simulink model "vit_tiny_simulink_v2"  (R2026a)
settings: Solver=FixedStepAuto
model workspace (MAT data in the model file):
  b0_bout = [0.778968453407 -0.0598331429064 0.518007159233 -0.0489355251193 -0.120259530842 -0.233932569623 0.145202979445 -0.609933674335 0.0480090156198 0.108426816761 -0.264556109905 0.0985349565744 ... (192 elements, 192x1)]
  b0_bqkv = [0.646790981293 1.11617136002 0.681947648525 0.380520701408 -0.228939250112 -0.210620775819 0.443939357996 0.168313741684 -0.0972141698003 0.138269111514 0.0691619515419 -0.232958853245 ... (576 elements, 576x1)]
  b0_wout = [-0.00644482811913 -0.0429702401161 0.0362374633551 -0.0623319223523 0.0345713756979 0.0856178253889 -0.087087020278 -0.0191561747342 -0.00712048402056 -0.00242649461143 -0.0159777421504 0.0877591148019 ... (36864 elements, 192x192)]
  b0_wqkv = [-0.111820198596 -0.061643447727 0.054388653487 -0.00236934260465 -0.124385409057 -0.0232415217906 -0.144188866019 0.097465723753 0.105003915727 -0.00340389972553 -0.123615674675 0.0953903868794 ... (110592 elements, 576x192)]
  b10_bout = [0.0401422604918 -0.173094883561 0.0153807215393 0.0737516358495 0.101318269968 -0.102552995086 -0.291259735823 -0.224377304316 0.0514300614595 -0.027960466221 -0.0377635993063 0.0201884657145 ... (192 elements, 192x1)]
  b10_bqkv = [-0.0802283063531 -0.193966910243 0.00535996071994 -0.170352563262 0.0527183525264 0.528374612331 0.381562888622 -0.241518482566 0.914053916931 -0.215931251645 0.12868578732 0.273458838463 ... (576 elements, 576x1)]
  b10_wout = [0.0782992020249 -0.0179750658572 0.129645258188 -0.0809217840433 0.0186945144087 0.0538404919207 -0.157687038183 -0.0309003889561 -0.0224377308041 -0.0750434324145 -0.134708985686 0.0406404659152 ... (36864 elements, 192x192)]
  b10_wqkv = [-0.0681082233787 0.0488544106483 -0.0731555297971 -0.0011171658989 -0.0821463242173 0.0175136197358 -0.0203201379627 0.0517607964575 -0.0496901981533 0.0509784445167 0.0563900731504 0.0606791935861 ... (110592 elements, 576x192)]
  b11_bout = [0.731424629688 -0.672566652298 0.280835986137 0.0499101057649 0.123574219644 0.0940038487315 -0.220306441188 -0.0221516657621 -0.404082298279 0.441653996706 0.00815785769373 0.0947464555502 ... (192 elements, 192x1)]
  b11_bqkv = [-0.18868714571 0.534532427788 -0.25027307868 -0.296293616295 -0.512328565121 -0.460017681122 -4.11578655243 0.164140149951 -1.2329877615 -0.281609892845 0.52692168951 0.468302696943 ... (576 elements, 576x1)]
  b11_wout = [0.047587364912 0.000814941420685 0.0597563162446 -0.0518249236047 -0.0508356280625 -0.0792408362031 -0.074274905026 -0.165682837367 -0.121390283108 0.0330879129469 -0.0537072867155 0.0743666738272 ... (36864 elements, 192x192)]
  b11_wqkv = [-0.0458272658288 0.0325416885316 0.0669981464744 0.0917598679662 0.0799371525645 -0.0330501869321 -0.000155119312694 0.0641588270664 0.144193008542 0.102804735303 0.00301717664115 0.0656418129802 ... (110592 elements, 576x192)]
  b1_bout = [-0.00971997715533 -0.00772349210456 -0.31704044342 0.238263577223 0.409832179546 0.283682137728 -0.0330189839005 -0.768799006939 -0.110244117677 -0.188653618097 -0.177126094699 0.0168285723776 ... (192 elements, 192x1)]
  b1_bqkv = [-0.44452881813 -0.77194994688 -0.318848788738 0.471629351377 -0.239391535521 -0.409112393856 -0.207066968083 0.650474190712 -0.0192827414721 0.194389820099 0.763711333275 -0.494900196791 ... (576 elements, 576x1)]
  b1_wout = [0.0807484909892 -0.00597395701334 -0.0312148649246 0.0136822443455 -0.03304643929 0.0206444934011 0.0792439058423 0.00468857167289 -0.025502268225 0.0522494241595 -0.0267972294241 -0.0434053950012 ... (36864 elements, 192x192)]
  b1_wqkv = [0.0512335635722 0.0885934457183 -0.00560034625232 0.00362458731979 -0.00930483732373 -0.0819085016847 0.0552942492068 -0.0290139894933 -0.0371151119471 -0.0522247776389 -0.0766076669097 -0.0357538573444 ... (110592 elements, 576x192)]
  b2_bout = [0.186909124255 -0.0196638796479 -0.0815877616405 -0.0520006269217 -0.0499247051775 -0.000725046906155 0.140784710646 -0.149694025517 0.0473203398287 0.0851504653692 -0.0695137456059 0.124424636364 ... (192 elements, 192x1)]
  b2_bqkv = [-0.0184468366206 -0.33409678936 0.0544146075845 -0.539437711239 0.0207475852221 -0.569913327694 0.675430297852 -0.0306138265878 0.137923911214 0.132708072662 -0.693137049675 -0.898855745792 ... (576 elements, 576x1)]
  b2_wout = [-0.0786214545369 -0.0264880564064 0.021444587037 -0.0203547999263 0.0681303590536 -0.0475320294499 -0.0878006964922 -0.117818422616 -0.0165466293693 -0.0438001379371 0.0672394111753 0.00145982671529 ... (36864 elements, 192x192)]
  b2_wqkv = [-0.138439133763 -0.164019718766 0.0208436828107 -0.0225581023842 0.0665642395616 0.0249229986221 -0.0014197943965 0.0313837975264 -0.118816934526 0.0859390795231 -0.0156196895987 0.0889494940639 ... (110592 elements, 576x192)]
  b3_bout = [0.0302332583815 -0.078589104116 0.0591629892588 0.122181482613 0.125220179558 0.174787655473 0.0698654949665 -0.119789279997 -0.0854825153947 0.0111166592687 -0.0519489310682 -0.0854979902506 ... (192 elements, 192x1)]
  b3_bqkv = [0.00970917753875 -0.137848749757 0.247470095754 0.00317194242962 -0.396429866552 -0.026160268113 -0.0386148132384 0.0218217838556 0.0342304669321 -0.283184826374 -0.23029743135 0.0519815944135 ... (576 elements, 576x1)]
  b3_wout = [-0.0932515189052 -0.0723011493683 -0.00572068244219 -0.0543470866978 -0.104117572308 -0.0373927094042 -0.0112823974341 0.0223814100027 0.0500929839909 -0.0560720935464 0.0953728854656 0.0339839868248 ... (36864 elements, 192x192)]
  b3_wqkv = [0.00368765974417 0.00305238785222 -0.026172157377 -0.0679289996624 -0.106250740588 -0.0884887948632 0.0913795605302 -0.0699781402946 -0.0334121137857 0.042931098491 0.0546709150076 -0.0805031657219 ... (110592 elements, 576x192)]
  b4_bout = [0.23899422586 -0.000535868050065 -0.0510758347809 0.0219226162881 -0.318580448627 0.0115311471745 0.197747603059 0.0553949847817 -0.137273982167 0.0953532159328 -0.0343873649836 -0.106459073722 ... (192 elements, 192x1)]
  b4_bqkv = [-0.0245674587786 0.118215315044 0.0117870233953 -0.434799820185 0.000942808925174 -0.0730947107077 -0.0735678821802 1.71554923058 0.0879050120711 -0.270164430141 -0.117167070508 -0.2562482059 ... (576 elements, 576x1)]
  b4_wout = [0.0284413192421 -0.0406549051404 -0.0393808446825 -0.0697678849101 -0.0218260530382 0.00707334280014 -0.0743876993656 -0.0783786103129 -0.0305015668273 0.093317463994 0.061121083796 -0.0506338961422 ... (36864 elements, 192x192)]
  b4_wqkv = [0.0929946824908 -0.0935411602259 -0.084877230227 -0.0577252544463 0.099425457418 -0.0690982490778 0.0233671385795 -0.0302681401372 0.0597265139222 0.00550593156368 -0.0276147350669 0.0846904069185 ... (110592 elements, 576x192)]
  b5_bout = [0.188547357917 0.024714069441 -0.0989287719131 0.223123833537 -0.177028283477 0.145788162947 0.310950517654 -0.0864898711443 0.122770495713 -0.125363752246 0.109310276806 -0.229095995426 ... (192 elements, 192x1)]
  b5_bqkv = [0.0722192823887 0.0234624873847 0.216983914375 -0.0264781974256 -0.159500107169 -0.0961934477091 0.0481547638774 0.0540090948343 0.0139488205314 0.0571327880025 0.021393366158 -0.0352926850319 ... (576 elements, 576x1)]
  b5_wout = [-0.0533635653555 -0.0483931489289 -0.00681060925126 -0.112240716815 -0.0576783418655 -0.0820446163416 -0.0322113111615 -0.0952084809542 0.0375515222549 -0.00923561304808 0.0461925640702 -0.0698399022222 ... (36864 elements, 192x192)]
  b5_wqkv = [0.0801055282354 0.0269768722355 -0.0491608083248 -0.0583752319217 0.0524758286774 -0.0386015921831 0.0413639172912 0.0464537888765 0.0647086799145 0.0535550974309 -0.110993593931 -0.00853264704347 ... (110592 elements, 576x192)]
  b6_bout = [0.112529121339 -0.0040144212544 0.0199170093983 -0.0523881353438 -0.25004824996 0.0551285110414 0.190843328834 0.185068517923 0.0474863760173 -0.0397604629397 -0.0546502992511 -0.194330573082 ... (192 elements, 192x1)]
  b6_bqkv = [-0.0338270738721 -2.49016571045 -0.199480056763 -0.0184682477266 -0.0240690167993 0.0215393863618 0.0194720793515 0.0613972917199 -0.0172453653067 0.0343660376966 0.0079789320007 0.00892005115747 ... (576 elements, 576x1)]
  b6_wout = [-0.0559973977506 -0.00380819896236 -0.0186422448605 -0.0984343886375 -0.130652368069 0.0328599624336 0.0286927837878 0.0624184831977 0.00975040718913 0.0448632091284 -0.0454246997833 -0.00807041116059 ... (36864 elements, 192x192)]
  b6_wqkv = [0.047971393913 -0.0175937246531 0.0648186728358 -0.0382851921022 0.0854341313243 0.0356842651963 -0.0363724976778 -0.0705645978451 0.0605219043791 -0.114698708057 -0.0194720495492 -0.0619053095579 ... (110592 elements, 576x192)]
  b7_bout = [-0.00979754887521 -0.196373164654 -0.0365842916071 0.0917368382215 0.095850341022 -0.0704315453768 -0.0610328689218 -0.103719733655 0.0393878184259 0.133041486144 0.156141653657 -0.306335449219 ... (192 elements, 192x1)]
  b7_bqkv = [0.11088873446 -0.0264491736889 0.0178037937731 0.0842389762402 -0.106872446835 -0.101556114852 -0.159019529819 -0.0193464122713 0.0995675623417 3.57965803146 0.0540712252259 -0.0657461732626 ... (576 elements, 576x1)]
  b7_wout = [-0.000260219909251 -0.033879969269 0.0518932491541 0.0383526273072 0.0138494819403 0.0156733635813 -0.0134258763865 0.00112146441825 -0.0264777150005 -0.0622148066759 -0.0190210156143 0.0761372745037 ... (36864 elements, 192x192)]
  b7_wqkv = [0.0141570502892 0.0168063379824 -0.0247797854245 0.0635327845812 -0.0980067625642 0.0770481750369 0.144644856453 -0.0683895945549 -0.0272835716605 0.0344916731119 0.0273532252759 -0.0550005435944 ... (110592 elements, 576x192)]
  b8_bout = [-0.0527804493904 0.117656506598 0.213438123465 0.106501460075 -0.0671964436769 0.0401020273566 0.060359813273 0.234292268753 0.0201724302024 -0.144064158201 0.0304094702005 -0.101683795452 ... (192 elements, 192x1)]
  b8_bqkv = [0.10499510169 0.0381473451853 0.129360511899 0.166689246893 0.0342182777822 0.174503937364 0.0605693124235 -0.618970632553 -0.0105152809992 -0.164437830448 -0.0311944670975 0.0511407628655 ... (576 elements, 576x1)]
  b8_wout = [-0.0634478852153 0.0849454700947 -0.023208366707 -0.00956540834159 -0.00672372383997 -0.0249805189669 -0.0135997496545 0.0273624900728 -0.0354879461229 0.0550138317049 0.134780943394 0.0173142403364 ... (36864 elements, 192x192)]
  b8_wqkv = [0.0237903837115 0.0325807631016 -0.0092166075483 0.049664542079 -0.0752728581429 -0.0574347376823 0.0816406607628 -0.00476910080761 -0.0516303107142 0.0683791711926 0.0430003665388 -0.0253584869206 ... (110592 elements, 576x192)]
  b9_bout = [0.0800115764141 -0.0413223616779 -0.181650936604 -0.0621207170188 0.0228195693344 0.10662330687 0.067500308156 -0.0102973049507 -0.0246045496315 0.00174500723369 -0.0965597853065 -0.0683078467846 ... (192 elements, 192x1)]
  b9_bqkv = [0.0875637978315 -0.020440755412 0.135997697711 0.0411557815969 0.0835343450308 0.2501488626 0.0407414548099 0.138864144683 0.0549810938537 -3.90157747269 -0.558510184288 0.620770931244 ... (576 elements, 576x1)]
  b9_wout = [0.0459634028375 -0.0737299397588 0.00984874274582 0.032143175602 0.00272075342946 0.00549572100863 0.047320663929 0.0298251267523 -0.0494017787278 -0.0186020601541 0.00333940866403 0.0266606453806 ... (36864 elements, 192x192)]
  b9_wqkv = [0.0156201086938 -0.0562715008855 0.205050706863 0.0245567578822 -0.0916293859482 0.0071588489227 -0.0374449826777 -0.0448822900653 -0.0624069795012 0.0729132145643 -0.0579476393759 -0.0632007867098 ... (110592 elements, 576x192)]
  cls_token_w = [-0.355721801519 -0.0376437492669 -0.0717144310474 0.0866070240736 0.02892735973 0.102299615741 -0.0759767442942 0.845572650433 0.0511999577284 -0.038422960788 0.122046947479 0.0408262424171 ... (192 elements, 192x1)]
  pos_embed_w = [-0.348548680544 -0.0297794546932 -0.0738909840584 0.0935556963086 0.0213174819946 0.127647742629 -0.0610382221639 0.847343623638 0.0437131524086 -0.0405866913497 0.121854871511 0.027680279687 ... (37824 elements, 192x197)]
values from neighbouring files (symbols this model uses):
  quantNet = quantize(quantObj)    [quantize_network.m]
libraries: dlconvfclib x26, dlnormalizationlib x25, dlcombinationlib x24, dlactivationlib x12
(part 2 of 3)

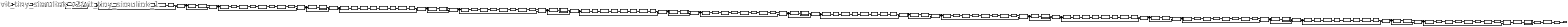
== system vit_tiny_simulink_v2/vit_tiny_simulink_1 ==
  input : Inport  OutDataTypeStr=single  PortDimensions="[224  224    3]"
  block0_add1 : dlcombinationlib/Addition Layer  NumInputs=2  OutMin=[]  OutMax=[]  InternalRndMeth=Floor  InternalSaturateOnIntegerOverflow=off  SampleTime=-1
      settings: DataFormat=CT
  block0_add2 : dlcombinationlib/Addition Layer  NumInputs=2  OutMin=[]  OutMax=[]  InternalRndMeth=Floor  InternalSaturateOnIntegerOverflow=off  SampleTime=-1
      settings: DataFormat=CT
  block0_attn : Subsystem
  block0_fc1 : dlconvfclib/Fully Connected Layer  Layer=quantNet.Layers(10)  OutMin=[]  OutMax=[]  OutDataTypeStr=fixdt(1,32,9)  RndMeth=Nearest  WeightsDataTypeStr=fixdt(1,8,7)  WeightsMin=[]  WeightsMax=[]  BiasDataTypeStr=fixdt(1,32,9)  BiasMin=[]  BiasMax=[]  AccumDataTypeStr=fixdt(1,32,9)  AccumMin=[]  AccumMax=[]  InternalRndMeth=Nearest  InternalSaturateOnIntegerOverflow=off  SampleTime=-1
      settings: DataFormat=CT
  block0_fc1_in_cast : DataTypeConversion  OutDataTypeStr=fixdt(1,8,2)  RndMeth=Nearest
  block0_fc1_out_cast : DataTypeConversion  OutDataTypeStr=fixdt('single')  RndMeth=Nearest
  block0_fc2 : dlconvfclib/Fully Connected Layer  Layer=quantNet.Layers(12)  OutMin=[]  OutMax=[]  OutDataTypeStr=fixdt(1,32,9)  RndMeth=Nearest  WeightsDataTypeStr=fixdt(1,8,7)  WeightsMin=[]  WeightsMax=[]  BiasDataTypeStr=fixdt(1,32,9)  BiasMin=[]  BiasMax=[]  AccumDataTypeStr=fixdt(1,32,9)  AccumMin=[]  AccumMax=[]  InternalRndMeth=Nearest  InternalSaturateOnIntegerOverflow=off  SampleTime=-1
      settings: DataFormat=CT
  block0_fc2_in_cast : DataTypeConversion  OutDataTypeStr=fixdt(1,8,2)  RndMeth=Nearest
  block0_fc2_out_cast : DataTypeConversion  OutDataTypeStr=fixdt('single')  RndMeth=Nearest
  block0_gelu : dlactivationlib/GELU Layer  Approximation=none  OutMin=[]  OutMax=[]  SampleTime=-1
  block0_ln1 : dlnormalizationlib/Layer Normalization Layer  Layer=quantNet.Layers(6)  OutMin=[]  OutMax=[]  ScaleDataTypeStr=Inherit: Inherit via back propagation  ScaleMin=[]  ScaleMax=[]  OffsetDataTypeStr=Inherit: Inherit via back propagation  OffsetMin=[]  OffsetMax=[]  SampleTime=-1
      settings: DataFormat=CT
  block0_ln2 : dlnormalizationlib/Layer Normalization Layer  Layer=quantNet.Layers(9)  OutMin=[]  OutMax=[]  ScaleDataTypeStr=Inherit: Inherit via back propagation  ScaleMin=[]  ScaleMax=[]  OffsetDataTypeStr=Inherit: Inherit via back propagation  OffsetMin=[]  OffsetMax=[]  SampleTime=-1
      settings: DataFormat=CT
  block10_add1 : dlcombinationlib/Addition Layer  NumInputs=2  OutMin=[]  OutMax=[]  InternalRndMeth=Floor  InternalSaturateOnIntegerOverflow=off  SampleTime=-1
      settings: DataFormat=CT
  block10_add2 : dlcombinationlib/Addition Layer  NumInputs=2  OutMin=[]  OutMax=[]  InternalRndMeth=Floor  InternalSaturateOnIntegerOverflow=off  SampleTime=-1
      settings: DataFormat=CT
  block10_attn : Subsystem
  block10_fc1 : dlconvfclib/Fully Connected Layer  Layer=quantNet.Layers(90)  OutMin=[]  OutMax=[]  OutDataTypeStr=fixdt(1,32,10)  RndMeth=Nearest  WeightsDataTypeStr=fixdt(1,8,7)  WeightsMin=[]  WeightsMax=[]  BiasDataTypeStr=fixdt(1,32,10)  BiasMin=[]  BiasMax=[]  AccumDataTypeStr=fixdt(1,32,10)  AccumMin=[]  AccumMax=[]  InternalRndMeth=Nearest  InternalSaturateOnIntegerOverflow=off  SampleTime=-1
      settings: DataFormat=CT
  block10_fc1_in_cast : DataTypeConversion  OutDataTypeStr=fixdt(1,8,3)  RndMeth=Nearest
  block10_fc1_out_cast : DataTypeConversion  OutDataTypeStr=fixdt('single')  RndMeth=Nearest
  block10_fc2 : dlconvfclib/Fully Connected Layer  Layer=quantNet.Layers(92)  OutMin=[]  OutMax=[]  OutDataTypeStr=fixdt(1,32,11)  RndMeth=Nearest  WeightsDataTypeStr=fixdt(1,8,7)  WeightsMin=[]  WeightsMax=[]  BiasDataTypeStr=fixdt(1,32,11)  BiasMin=[]  BiasMax=[]  AccumDataTypeStr=fixdt(1,32,11)  AccumMin=[]  AccumMax=[]  InternalRndMeth=Nearest  InternalSaturateOnIntegerOverflow=off  SampleTime=-1
      settings: DataFormat=CT
  block10_fc2_in_cast : DataTypeConversion  OutDataTypeStr=fixdt(1,8,4)  RndMeth=Nearest
  block10_fc2_out_cast : DataTypeConversion  OutDataTypeStr=fixdt('single')  RndMeth=Nearest
  block10_gelu : dlactivationlib/GELU Layer  Approximation=none  OutMin=[]  OutMax=[]  SampleTime=-1
  block10_ln1 : dlnormalizationlib/Layer Normalization Layer  Layer=quantNet.Layers(86)  OutMin=[]  OutMax=[]  ScaleDataTypeStr=Inherit: Inherit via back propagation  ScaleMin=[]  ScaleMax=[]  OffsetDataTypeStr=Inherit: Inherit via back propagation  OffsetMin=[]  OffsetMax=[]  SampleTime=-1
      settings: DataFormat=CT
  block10_ln2 : dlnormalizationlib/Layer Normalization Layer  Layer=quantNet.Layers(89)  OutMin=[]  OutMax=[]  ScaleDataTypeStr=Inherit: Inherit via back propagation  ScaleMin=[]  ScaleMax=[]  OffsetDataTypeStr=Inherit: Inherit via back propagation  OffsetMin=[]  OffsetMax=[]  SampleTime=-1
      settings: DataFormat=CT
  block11_add1 : dlcombinationlib/Addition Layer  NumInputs=2  OutMin=[]  OutMax=[]  InternalRndMeth=Floor  InternalSaturateOnIntegerOverflow=off  SampleTime=-1
      settings: DataFormat=CT
  block11_add2 : dlcombinationlib/Addition Layer  NumInputs=2  OutMin=[]  OutMax=[]  InternalRndMeth=Floor  InternalSaturateOnIntegerOverflow=off  SampleTime=-1
      settings: DataFormat=CT
  block11_attn : Subsystem
  block11_fc1 : dlconvfclib/Fully Connected Layer  Layer=quantNet.Layers(98)  OutMin=[]  OutMax=[]  OutDataTypeStr=fixdt(1,32,11)  RndMeth=Nearest  WeightsDataTypeStr=fixdt(1,8,8)  WeightsMin=[]  WeightsMax=[]  BiasDataTypeStr=fixdt(1,32,11)  BiasMin=[]  BiasMax=[]  AccumDataTypeStr=fixdt(1,32,11)  AccumMin=[]  AccumMax=[]  InternalRndMeth=Nearest  InternalSaturateOnIntegerOverflow=off  SampleTime=-1
      settings: DataFormat=CT
  block11_fc1_in_cast : DataTypeConversion  OutDataTypeStr=fixdt(1,8,3)  RndMeth=Nearest
  block11_fc1_out_cast : DataTypeConversion  OutDataTypeStr=fixdt('single')  RndMeth=Nearest
  block11_fc2 : dlconvfclib/Fully Connected Layer  Layer=quantNet.Layers(100)  OutMin=[]  OutMax=[]  OutDataTypeStr=fixdt(1,32,11)  RndMeth=Nearest  WeightsDataTypeStr=fixdt(1,8,7)  WeightsMin=[]  WeightsMax=[]  BiasDataTypeStr=fixdt(1,32,11)  BiasMin=[]  BiasMax=[]  AccumDataTypeStr=fixdt(1,32,11)  AccumMin=[]  AccumMax=[]  InternalRndMeth=Nearest  InternalSaturateOnIntegerOverflow=off  SampleTime=-1
      settings: DataFormat=CT
  block11_fc2_in_cast : DataTypeConversion  OutDataTypeStr=fixdt(1,8,4)  RndMeth=Nearest
  block11_fc2_out_cast : DataTypeConversion  OutDataTypeStr=fixdt('single')  RndMeth=Nearest
  block11_gelu : dlactivationlib/GELU Layer  Approximation=none  OutMin=[]  OutMax=[]  SampleTime=-1
  block11_ln1 : dlnormalizationlib/Layer Normalization Layer  Layer=quantNet.Layers(94)  OutMin=[]  OutMax=[]  ScaleDataTypeStr=Inherit: Inherit via back propagation  ScaleMin=[]  ScaleMax=[]  OffsetDataTypeStr=Inherit: Inherit via back propagation  OffsetMin=[]  OffsetMax=[]  SampleTime=-1
      settings: DataFormat=CT
  block11_ln2 : dlnormalizationlib/Layer Normalization Layer  Layer=quantNet.Layers(97)  OutMin=[]  OutMax=[]  ScaleDataTypeStr=Inherit: Inherit via back propagation  ScaleMin=[]  ScaleMax=[]  OffsetDataTypeStr=Inherit: Inherit via back propagation  OffsetMin=[]  OffsetMax=[]  SampleTime=-1
      settings: DataFormat=CT
  block1_add1 : dlcombinationlib/Addition Layer  NumInputs=2  OutMin=[]  OutMax=[]  InternalRndMeth=Floor  InternalSaturateOnIntegerOverflow=off  SampleTime=-1
      settings: DataFormat=CT
  block1_add2 : dlcombinationlib/Addition Layer  NumInputs=2  OutMin=[]  OutMax=[]  InternalRndMeth=Floor  InternalSaturateOnIntegerOverflow=off  SampleTime=-1
      settings: DataFormat=CT
  block1_attn : Subsystem
  block1_fc1 : dlconvfclib/Fully Connected Layer  Layer=quantNet.Layers(18)  OutMin=[]  OutMax=[]  OutDataTypeStr=fixdt(1,32,10)  RndMeth=Nearest  WeightsDataTypeStr=fixdt(1,8,7)  WeightsMin=[]  WeightsMax=[]  BiasDataTypeStr=fixdt(1,32,10)  BiasMin=[]  BiasMax=[]  AccumDataTypeStr=fixdt(1,32,10)  AccumMin=[]  AccumMax=[]  InternalRndMeth=Nearest  InternalSaturateOnIntegerOverflow=off  SampleTime=-1
      settings: DataFormat=CT
  block1_fc1_in_cast : DataTypeConversion  OutDataTypeStr=fixdt(1,8,3)  RndMeth=Nearest
  block1_fc1_out_cast : DataTypeConversion  OutDataTypeStr=fixdt('single')  RndMeth=Nearest
  block1_fc2 : dlconvfclib/Fully Connected Layer  Layer=quantNet.Layers(20)  OutMin=[]  OutMax=[]  OutDataTypeStr=fixdt(1,32,10)  RndMeth=Nearest  WeightsDataTypeStr=fixdt(1,8,7)  WeightsMin=[]  WeightsMax=[]  BiasDataTypeStr=fixdt(1,32,10)  BiasMin=[]  BiasMax=[]  AccumDataTypeStr=fixdt(1,32,10)  AccumMin=[]  AccumMax=[]  InternalRndMeth=Nearest  InternalSaturateOnIntegerOverflow=off  SampleTime=-1
      settings: DataFormat=CT
  block1_fc2_in_cast : DataTypeConversion  OutDataTypeStr=fixdt(1,8,3)  RndMeth=Nearest
  block1_fc2_out_cast : DataTypeConversion  OutDataTypeStr=fixdt('single')  RndMeth=Nearest
  block1_gelu : dlactivationlib/GELU Layer  Approximation=none  OutMin=[]  OutMax=[]  SampleTime=-1
  block1_ln1 : dlnormalizationlib/Layer Normalization Layer  Layer=quantNet.Layers(14)  OutMin=[]  OutMax=[]  ScaleDataTypeStr=Inherit: Inherit via back propagation  ScaleMin=[]  ScaleMax=[]  OffsetDataTypeStr=Inherit: Inherit via back propagation  OffsetMin=[]  OffsetMax=[]  SampleTime=-1
      settings: DataFormat=CT
  block1_ln2 : dlnormalizationlib/Layer Normalization Layer  Layer=quantNet.Layers(17)  OutMin=[]  OutMax=[]  ScaleDataTypeStr=Inherit: Inherit via back propagation  ScaleMin=[]  ScaleMax=[]  OffsetDataTypeStr=Inherit: Inherit via back propagation  OffsetMin=[]  OffsetMax=[]  SampleTime=-1
      settings: DataFormat=CT
  block2_add1 : dlcombinationlib/Addition Layer  NumInputs=2  OutMin=[]  OutMax=[]  InternalRndMeth=Floor  InternalSaturateOnIntegerOverflow=off  SampleTime=-1
      settings: DataFormat=CT
  block2_add2 : dlcombinationlib/Addition Layer  NumInputs=2  OutMin=[]  OutMax=[]  InternalRndMeth=Floor  InternalSaturateOnIntegerOverflow=off  SampleTime=-1
      settings: DataFormat=CT
  block2_attn : Subsystem
  block2_fc1 : dlconvfclib/Fully Connected Layer  Layer=quantNet.Layers(26)  OutMin=[]  OutMax=[]  OutDataTypeStr=fixdt(1,32,10)  RndMeth=Nearest  WeightsDataTypeStr=fixdt(1,8,7)  WeightsMin=[]  WeightsMax=[]  BiasDataTypeStr=fixdt(1,32,10)  BiasMin=[]  BiasMax=[]  AccumDataTypeStr=fixdt(1,32,10)  AccumMin=[]  AccumMax=[]  InternalRndMeth=Nearest  InternalSaturateOnIntegerOverflow=off  SampleTime=-1
      settings: DataFormat=CT
  block2_fc1_in_cast : DataTypeConversion  OutDataTypeStr=fixdt(1,8,3)  RndMeth=Nearest
  block2_fc1_out_cast : DataTypeConversion  OutDataTypeStr=fixdt('single')  RndMeth=Nearest
  block2_fc2 : dlconvfclib/Fully Connected Layer  Layer=quantNet.Layers(28)  OutMin=[]  OutMax=[]  OutDataTypeStr=fixdt(1,32,10)  RndMeth=Nearest  WeightsDataTypeStr=fixdt(1,8,7)  WeightsMin=[]  WeightsMax=[]  BiasDataTypeStr=fixdt(1,32,10)  BiasMin=[]  BiasMax=[]  AccumDataTypeStr=fixdt(1,32,10)  AccumMin=[]  AccumMax=[]  InternalRndMeth=Nearest  InternalSaturateOnIntegerOverflow=off  SampleTime=-1
      settings: DataFormat=CT
  block2_fc2_in_cast : DataTypeConversion  OutDataTypeStr=fixdt(1,8,3)  RndMeth=Nearest
  block2_fc2_out_cast : DataTypeConversion  OutDataTypeStr=fixdt('single')  RndMeth=Nearest
  block2_gelu : dlactivationlib/GELU Layer  Approximation=none  OutMin=[]  OutMax=[]  SampleTime=-1
  block2_ln1 : dlnormalizationlib/Layer Normalization Layer  Layer=quantNet.Layers(22)  OutMin=[]  OutMax=[]  ScaleDataTypeStr=Inherit: Inherit via back propagation  ScaleMin=[]  ScaleMax=[]  OffsetDataTypeStr=Inherit: Inherit via back propagation  OffsetMin=[]  OffsetMax=[]  SampleTime=-1
      settings: DataFormat=CT
  block2_ln2 : dlnormalizationlib/Layer Normalization Layer  Layer=quantNet.Layers(25)  OutMin=[]  OutMax=[]  ScaleDataTypeStr=Inherit: Inherit via back propagation  ScaleMin=[]  ScaleMax=[]  OffsetDataTypeStr=Inherit: Inherit via back propagation  OffsetMin=[]  OffsetMax=[]  SampleTime=-1
      settings: DataFormat=CT
  block3_add1 : dlcombinationlib/Addition Layer  NumInputs=2  OutMin=[]  OutMax=[]  InternalRndMeth=Floor  InternalSaturateOnIntegerOverflow=off  SampleTime=-1
      settings: DataFormat=CT
  block3_add2 : dlcombinationlib/Addition Layer  NumInputs=2  OutMin=[]  OutMax=[]  InternalRndMeth=Floor  InternalSaturateOnIntegerOverflow=off  SampleTime=-1
      settings: DataFormat=CT
  block3_attn : Subsystem
  block3_fc1 : dlconvfclib/Fully Connected Layer  Layer=quantNet.Layers(34)  OutMin=[]  OutMax=[]  OutDataTypeStr=fixdt(1,32,10)  RndMeth=Nearest  WeightsDataTypeStr=fixdt(1,8,7)  WeightsMin=[]  WeightsMax=[]  BiasDataTypeStr=fixdt(1,32,10)  BiasMin=[]  BiasMax=[]  AccumDataTypeStr=fixdt(1,32,10)  AccumMin=[]  AccumMax=[]  InternalRndMeth=Nearest  InternalSaturateOnIntegerOverflow=off  SampleTime=-1
      settings: DataFormat=CT
  block3_fc1_in_cast : DataTypeConversion  OutDataTypeStr=fixdt(1,8,3)  RndMeth=Nearest
  block3_fc1_out_cast : DataTypeConversion  OutDataTypeStr=fixdt('single')  RndMeth=Nearest
  block3_fc2 : dlconvfclib/Fully Connected Layer  Layer=quantNet.Layers(36)  OutMin=[]  OutMax=[]  OutDataTypeStr=fixdt(1,32,11)  RndMeth=Nearest  WeightsDataTypeStr=fixdt(1,8,7)  WeightsMin=[]  WeightsMax=[]  BiasDataTypeStr=fixdt(1,32,11)  BiasMin=[]  BiasMax=[]  AccumDataTypeStr=fixdt(1,32,11)  AccumMin=[]  AccumMax=[]  InternalRndMeth=Nearest  InternalSaturateOnIntegerOverflow=off  SampleTime=-1
      settings: DataFormat=CT
  block3_fc2_in_cast : DataTypeConversion  OutDataTypeStr=fixdt(1,8,4)  RndMeth=Nearest
  block3_fc2_out_cast : DataTypeConversion  OutDataTypeStr=fixdt('single')  RndMeth=Nearest
  block3_gelu : dlactivationlib/GELU Layer  Approximation=none  OutMin=[]  OutMax=[]  SampleTime=-1
  block3_ln1 : dlnormalizationlib/Layer Normalization Layer  Layer=quantNet.Layers(30)  OutMin=[]  OutMax=[]  ScaleDataTypeStr=Inherit: Inherit via back propagation  ScaleMin=[]  ScaleMax=[]  OffsetDataTypeStr=Inherit: Inherit via back propagation  OffsetMin=[]  OffsetMax=[]  SampleTime=-1
      settings: DataFormat=CT
  block3_ln2 : dlnormalizationlib/Layer Normalization Layer  Layer=quantNet.Layers(33)  OutMin=[]  OutMax=[]  ScaleDataTypeStr=Inherit: Inherit via back propagation  ScaleMin=[]  ScaleMax=[]  OffsetDataTypeStr=Inherit: Inherit via back propagation  OffsetMin=[]  OffsetMax=[]  SampleTime=-1
      settings: DataFormat=CT
  block4_add1 : dlcombinationlib/Addition Layer  NumInputs=2  OutMin=[]  OutMax=[]  InternalRndMeth=Floor  InternalSaturateOnIntegerOverflow=off  SampleTime=-1
      settings: DataFormat=CT
  block4_add2 : dlcombinationlib/Addition Layer  NumInputs=2  OutMin=[]  OutMax=[]  InternalRndMeth=Floor  InternalSaturateOnIntegerOverflow=off  SampleTime=-1
      settings: DataFormat=CT
  block4_attn : Subsystem
  block4_fc1 : dlconvfclib/Fully Connected Layer  Layer=quantNet.Layers(42)  OutMin=[]  OutMax=[]  OutDataTypeStr=fixdt(1,32,12)  RndMeth=Nearest  WeightsDataTypeStr=fixdt(1,8,8)  WeightsMin=[]  WeightsMax=[]  BiasDataTypeStr=fixdt(1,32,12)  BiasMin=[]  BiasMax=[]  AccumDataTypeStr=fixdt(1,32,12)  AccumMin=[]  AccumMax=[]  InternalRndMeth=Nearest  InternalSaturateOnIntegerOverflow=off  SampleTime=-1
      settings: DataFormat=CT
  block4_fc1_in_cast : DataTypeConversion  OutDataTypeStr=fixdt(1,8,4)  RndMeth=Nearest
  block4_fc1_out_cast : DataTypeConversion  OutDataTypeStr=fixdt('single')  RndMeth=Nearest
  block4_fc2 : dlconvfclib/Fully Connected Layer  Layer=quantNet.Layers(44)  OutMin=[]  OutMax=[]  OutDataTypeStr=fixdt(1,32,12)  RndMeth=Nearest  WeightsDataTypeStr=fixdt(1,8,8)  WeightsMin=[]  WeightsMax=[]  BiasDataTypeStr=fixdt(1,32,12)  BiasMin=[]  BiasMax=[]  AccumDataTypeStr=fixdt(1,32,12)  AccumMin=[]  AccumMax=[]  InternalRndMeth=Nearest  InternalSaturateOnIntegerOverflow=off  SampleTime=-1
      settings: DataFormat=CT
  block4_fc2_in_cast : DataTypeConversion  OutDataTypeStr=fixdt(1,8,4)  RndMeth=Nearest
  block4_fc2_out_cast : DataTypeConversion  OutDataTypeStr=fixdt('single')  RndMeth=Nearest
  block4_gelu : dlactivationlib/GELU Layer  Approximation=none  OutMin=[]  OutMax=[]  SampleTime=-1
  block4_ln1 : dlnormalizationlib/Layer Normalization Layer  Layer=quantNet.Layers(38)  OutMin=[]  OutMax=[]  ScaleDataTypeStr=Inherit: Inherit via back propagation  ScaleMin=[]  ScaleMax=[]  OffsetDataTypeStr=Inherit: Inherit via back propagation  OffsetMin=[]  OffsetMax=[]  SampleTime=-1
      settings: DataFormat=CT
  block4_ln2 : dlnormalizationlib/Layer Normalization Layer  Layer=quantNet.Layers(41)  OutMin=[]  OutMax=[]  ScaleDataTypeStr=Inherit: Inherit via back propagation  ScaleMin=[]  ScaleMax=[]  OffsetDataTypeStr=Inherit: Inherit via back propagation  OffsetMin=[]  OffsetMax=[]  SampleTime=-1
      settings: DataFormat=CT
  block5_add1 : dlcombinationlib/Addition Layer  NumInputs=2  OutMin=[]  OutMax=[]  InternalRndMeth=Floor  InternalSaturateOnIntegerOverflow=off  SampleTime=-1
      settings: DataFormat=CT
  block5_add2 : dlcombinationlib/Addition Layer  NumInputs=2  OutMin=[]  OutMax=[]  InternalRndMeth=Floor  InternalSaturateOnIntegerOverflow=off  SampleTime=-1
      settings: DataFormat=CT
  block5_attn : Subsystem
  block5_fc1 : dlconvfclib/Fully Connected Layer  Layer=quantNet.Layers(50)  OutMin=[]  OutMax=[]  OutDataTypeStr=fixdt(1,32,12)  RndMeth=Nearest  WeightsDataTypeStr=fixdt(1,8,8)  WeightsMin=[]  WeightsMax=[]  BiasDataTypeStr=fixdt(1,32,12)  BiasMin=[]  BiasMax=[]  AccumDataTypeStr=fixdt(1,32,12)  AccumMin=[]  AccumMax=[]  InternalRndMeth=Nearest  InternalSaturateOnIntegerOverflow=off  SampleTime=-1
      settings: DataFormat=CT
  block5_fc1_in_cast : DataTypeConversion  OutDataTypeStr=fixdt(1,8,4)  RndMeth=Nearest
  block5_fc1_out_cast : DataTypeConversion  OutDataTypeStr=fixdt('single')  RndMeth=Nearest
  block5_fc2 : dlconvfclib/Fully Connected Layer  Layer=quantNet.Layers(52)  OutMin=[]  OutMax=[]  OutDataTypeStr=fixdt(1,32,12)  RndMeth=Nearest  WeightsDataTypeStr=fixdt(1,8,8)  WeightsMin=[]  WeightsMax=[]  BiasDataTypeStr=fixdt(1,32,12)  BiasMin=[]  BiasMax=[]  AccumDataTypeStr=fixdt(1,32,12)  AccumMin=[]  AccumMax=[]  InternalRndMeth=Nearest  InternalSaturateOnIntegerOverflow=off  SampleTime=-1
      settings: DataFormat=CT
  block5_fc2_in_cast : DataTypeConversion  OutDataTypeStr=fixdt(1,8,4)  RndMeth=Nearest
  block5_fc2_out_cast : DataTypeConversion  OutDataTypeStr=fixdt('single')  RndMeth=Nearest
  block5_gelu : dlactivationlib/GELU Layer  Approximation=none  OutMin=[]  OutMax=[]  SampleTime=-1
  block5_ln1 : dlnormalizationlib/Layer Normalization Layer  Layer=quantNet.Layers(46)  OutMin=[]  OutMax=[]  ScaleDataTypeStr=Inherit: Inherit via back propagation  ScaleMin=[]  ScaleMax=[]  OffsetDataTypeStr=Inherit: Inherit via back propagation  OffsetMin=[]  OffsetMax=[]  SampleTime=-1
      settings: DataFormat=CT
  block5_ln2 : dlnormalizationlib/Layer Normalization Layer  Layer=quantNet.Layers(49)  OutMin=[]  OutMax=[]  ScaleDataTypeStr=Inherit: Inherit via back propagation  ScaleMin=[]  ScaleMax=[]  OffsetDataTypeStr=Inherit: Inherit via back propagation  OffsetMin=[]  OffsetMax=[]  SampleTime=-1
      settings: DataFormat=CT
  block6_add1 : dlcombinationlib/Addition Layer  NumInputs=2  OutMin=[]  OutMax=[]  InternalRndMeth=Floor  InternalSaturateOnIntegerOverflow=off  SampleTime=-1
      settings: DataFormat=CT
  block6_add2 : dlcombinationlib/Addition Layer  NumInputs=2  OutMin=[]  OutMax=[]  InternalRndMeth=Floor  InternalSaturateOnIntegerOverflow=off  SampleTime=-1
      settings: DataFormat=CT
  block6_attn : Subsystem
  block6_fc1 : dlconvfclib/Fully Connected Layer  Layer=quantNet.Layers(58)  OutMin=[]  OutMax=[]  OutDataTypeStr=fixdt(1,32,12)  RndMeth=Nearest  WeightsDataTypeStr=fixdt(1,8,8)  WeightsMin=[]  WeightsMax=[]  BiasDataTypeStr=fixdt(1,32,12)  BiasMin=[]  BiasMax=[]  AccumDataTypeStr=fixdt(1,32,12)  AccumMin=[]  AccumMax=[]  InternalRndMeth=Nearest  InternalSaturateOnIntegerOverflow=off  SampleTime=-1
      settings: DataFormat=CT
  block6_fc1_in_cast : DataTypeConversion  OutDataTypeStr=fixdt(1,8,4)  RndMeth=Nearest
  block6_fc1_out_cast : DataTypeConversion  OutDataTypeStr=fixdt('single')  RndMeth=Nearest
  block6_fc2 : dlconvfclib/Fully Connected Layer  Layer=quantNet.Layers(60)  OutMin=[]  OutMax=[]  OutDataTypeStr=fixdt(1,32,10)  RndMeth=Nearest  WeightsDataTypeStr=fixdt(1,8,7)  WeightsMin=[]  WeightsMax=[]  BiasDataTypeStr=fixdt(1,32,10)  BiasMin=[]  BiasMax=[]  AccumDataTypeStr=fixdt(1,32,10)  AccumMin=[]  AccumMax=[]  InternalRndMeth=Nearest  InternalSaturateOnIntegerOverflow=off  SampleTime=-1
      settings: DataFormat=CT
  block6_fc2_in_cast : DataTypeConversion  OutDataTypeStr=fixdt(1,8,3)  RndMeth=Nearest
  block6_fc2_out_cast : DataTypeConversion  OutDataTypeStr=fixdt('single')  RndMeth=Nearest
  block6_gelu : dlactivationlib/GELU Layer  Approximation=none  OutMin=[]  OutMax=[]  SampleTime=-1
  block6_ln1 : dlnormalizationlib/Layer Normalization Layer  Layer=quantNet.Layers(54)  OutMin=[]  OutMax=[]  ScaleDataTypeStr=Inherit: Inherit via back propagation  ScaleMin=[]  ScaleMax=[]  OffsetDataTypeStr=Inherit: Inherit via back propagation  OffsetMin=[]  OffsetMax=[]  SampleTime=-1
      settings: DataFormat=CT
  block6_ln2 : dlnormalizationlib/Layer Normalization Layer  Layer=quantNet.Layers(57)  OutMin=[]  OutMax=[]  ScaleDataTypeStr=Inherit: Inherit via back propagation  ScaleMin=[]  ScaleMax=[]  OffsetDataTypeStr=Inherit: Inherit via back propagation  OffsetMin=[]  OffsetMax=[]  SampleTime=-1
      settings: DataFormat=CT
  block7_add1 : dlcombinationlib/Addition Layer  NumInputs=2  OutMin=[]  OutMax=[]  InternalRndMeth=Floor  InternalSaturateOnIntegerOverflow=off  SampleTime=-1
      settings: DataFormat=CT
  block7_add2 : dlcombinationlib/Addition Layer  NumInputs=2  OutMin=[]  OutMax=[]  InternalRndMeth=Floor  InternalSaturateOnIntegerOverflow=off  SampleTime=-1
      settings: DataFormat=CT
  block7_attn : Subsystem
  block7_fc1 : dlconvfclib/Fully Connected Layer  Layer=quantNet.Layers(66)  OutMin=[]  OutMax=[]  OutDataTypeStr=fixdt(1,32,9)  RndMeth=Nearest  WeightsDataTypeStr=fixdt(1,8,5)  WeightsMin=[]  WeightsMax=[]  BiasDataTypeStr=fixdt(1,32,9)  BiasMin=[]  BiasMax=[]  AccumDataTypeStr=fixdt(1,32,9)  AccumMin=[]  AccumMax=[]  InternalRndMeth=Nearest  InternalSaturateOnIntegerOverflow=off  SampleTime=-1
      settings: DataFormat=CT
  block7_fc1_in_cast : DataTypeConversion  OutDataTypeStr=fixdt(1,8,4)  RndMeth=Nearest
  block7_fc1_out_cast : DataTypeConversion  OutDataTypeStr=fixdt('single')  RndMeth=Nearest
  block7_fc2 : dlconvfclib/Fully Connected Layer  Layer=quantNet.Layers(68)  OutMin=[]  OutMax=[]  OutDataTypeStr=fixdt(1,32,4)  RndMeth=Nearest  WeightsDataTypeStr=fixdt(1,8,5)  WeightsMin=[]  WeightsMax=[]  BiasDataTypeStr=fixdt(1,32,4)  BiasMin=[]  BiasMax=[]  AccumDataTypeStr=fixdt(1,32,4)  AccumMin=[]  AccumMax=[]  InternalRndMeth=Nearest  InternalSaturateOnIntegerOverflow=off  SampleTime=-1
      settings: DataFormat=CT
  block7_fc2_in_cast : DataTypeConversion  OutDataTypeStr=fixdt(1,8,-1)  RndMeth=Nearest
  block7_fc2_out_cast : DataTypeConversion  OutDataTypeStr=fixdt('single')  RndMeth=Nearest
  block7_gelu : dlactivationlib/GELU Layer  Approximation=none  OutMin=[]  OutMax=[]  SampleTime=-1
  block7_ln1 : dlnormalizationlib/Layer Normalization Layer  Layer=quantNet.Layers(62)  OutMin=[]  OutMax=[]  ScaleDataTypeStr=Inherit: Inherit via back propagation  ScaleMin=[]  ScaleMax=[]  OffsetDataTypeStr=Inherit: Inherit via back propagation  OffsetMin=[]  OffsetMax=[]  SampleTime=-1
      settings: DataFormat=CT
  block7_ln2 : dlnormalizationlib/Layer Normalization Layer  Layer=quantNet.Layers(65)  OutMin=[]  OutMax=[]  ScaleDataTypeStr=Inherit: Inherit via back propagation  ScaleMin=[]  ScaleMax=[]  OffsetDataTypeStr=Inherit: Inherit via back propagation  OffsetMin=[]  OffsetMax=[]  SampleTime=-1
      settings: DataFormat=CT
  block8_add1 : dlcombinationlib/Addition Layer  NumInputs=2  OutMin=[]  OutMax=[]  InternalRndMeth=Floor  InternalSaturateOnIntegerOverflow=off  SampleTime=-1
      settings: DataFormat=CT
  block8_add2 : dlcombinationlib/Addition Layer  NumInputs=2  OutMin=[]  OutMax=[]  InternalRndMeth=Floor  InternalSaturateOnIntegerOverflow=off  SampleTime=-1
      settings: DataFormat=CT
  block8_attn : Subsystem
  block8_fc1 : dlconvfclib/Fully Connected Layer  Layer=quantNet.Layers(74)  OutMin=[]  OutMax=[]  OutDataTypeStr=fixdt(1,32,11)  RndMeth=Nearest  WeightsDataTypeStr=fixdt(1,8,8)  WeightsMin=[]  WeightsMax=[]  BiasDataTypeStr=fixdt(1,32,11)  BiasMin=[]  BiasMax=[]  AccumDataTypeStr=fixdt(1,32,11)  AccumMin=[]  AccumMax=[]  InternalRndMeth=Nearest  InternalSaturateOnIntegerOverflow=off  SampleTime=-1
      settings: DataFormat=CT
  block8_fc1_in_cast : DataTypeConversion  OutDataTypeStr=fixdt(1,8,3)  RndMeth=Nearest
  block8_fc1_out_cast : DataTypeConversion  OutDataTypeStr=fixdt('single')  RndMeth=Nearest
  block8_fc2 : dlconvfclib/Fully Connected Layer  Layer=quantNet.Layers(76)  OutMin=[]  OutMax=[]  OutDataTypeStr=fixdt(1,32,11)  RndMeth=Nearest  WeightsDataTypeStr=fixdt(1,8,7)  WeightsMin=[]  WeightsMax=[]  BiasDataTypeStr=fixdt(1,32,11)  BiasMin=[]  BiasMax=[]  AccumDataTypeStr=fixdt(1,32,11)  AccumMin=[]  AccumMax=[]  InternalRndMeth=Nearest  InternalSaturateOnIntegerOverflow=off  SampleTime=-1
      settings: DataFormat=CT
  block8_fc2_in_cast : DataTypeConversion  OutDataTypeStr=fixdt(1,8,4)  RndMeth=Nearest
  block8_fc2_out_cast : DataTypeConversion  OutDataTypeStr=fixdt('single')  RndMeth=Nearest
  block8_gelu : dlactivationlib/GELU Layer  Approximation=none  OutMin=[]  OutMax=[]  SampleTime=-1
  block8_ln1 : dlnormalizationlib/Layer Normalization Layer  Layer=quantNet.Layers(70)  OutMin=[]  OutMax=[]  ScaleDataTypeStr=Inherit: Inherit via back propagation  ScaleMin=[]  ScaleMax=[]  OffsetDataTypeStr=Inherit: Inherit via back propagation  OffsetMin=[]  OffsetMax=[]  SampleTime=-1
      settings: DataFormat=CT
  block8_ln2 : dlnormalizationlib/Layer Normalization Layer  Layer=quantNet.Layers(73)  OutMin=[]  OutMax=[]  ScaleDataTypeStr=Inherit: Inherit via back propagation  ScaleMin=[]  ScaleMax=[]  OffsetDataTypeStr=Inherit: Inherit via back propagation  OffsetMin=[]  OffsetMax=[]  SampleTime=-1
      settings: DataFormat=CT
  block9_add1 : dlcombinationlib/Addition Layer  NumInputs=2  OutMin=[]  OutMax=[]  InternalRndMeth=Floor  InternalSaturateOnIntegerOverflow=off  SampleTime=-1
      settings: DataFormat=CT
  block9_add2 : dlcombinationlib/Addition Layer  NumInputs=2  OutMin=[]  OutMax=[]  InternalRndMeth=Floor  InternalSaturateOnIntegerOverflow=off  SampleTime=-1
      settings: DataFormat=CT
  block9_attn : Subsystem
  block9_fc1 : dlconvfclib/Fully Connected Layer  Layer=quantNet.Layers(82)  OutMin=[]  OutMax=[]  OutDataTypeStr=fixdt(1,32,10)  RndMeth=Nearest  WeightsDataTypeStr=fixdt(1,8,7)  WeightsMin=[]  WeightsMax=[]  BiasDataTypeStr=fixdt(1,32,10)  BiasMin=[]  BiasMax=[]  AccumDataTypeStr=fixdt(1,32,10)  AccumMin=[]  AccumMax=[]  InternalRndMeth=Nearest  InternalSaturateOnIntegerOverflow=off  SampleTime=-1
      settings: DataFormat=CT
  block9_fc1_in_cast : DataTypeConversion  OutDataTypeStr=fixdt(1,8,3)  RndMeth=Nearest
  block9_fc1_out_cast : DataTypeConversion  OutDataTypeStr=fixdt('single')  RndMeth=Nearest
  block9_fc2 : dlconvfclib/Fully Connected Layer  Layer=quantNet.Layers(84)  OutMin=[]  OutMax=[]  OutDataTypeStr=fixdt(1,32,11)  RndMeth=Nearest  WeightsDataTypeStr=fixdt(1,8,7)  WeightsMin=[]  WeightsMax=[]  BiasDataTypeStr=fixdt(1,32,11)  BiasMin=[]  BiasMax=[]  AccumDataTypeStr=fixdt(1,32,11)  AccumMin=[]  AccumMax=[]  InternalRndMeth=Nearest  InternalSaturateOnIntegerOverflow=off  SampleTime=-1
      settings: DataFormat=CT
  block9_fc2_in_cast : DataTypeConversion  OutDataTypeStr=fixdt(1,8,4)  RndMeth=Nearest
  block9_fc2_out_cast : DataTypeConversion  OutDataTypeStr=fixdt('single')  RndMeth=Nearest
  block9_gelu : dlactivationlib/GELU Layer  Approximation=none  OutMin=[]  OutMax=[]  SampleTime=-1
  block9_ln1 : dlnormalizationlib/Layer Normalization Layer  Layer=quantNet.Layers(78)  OutMin=[]  OutMax=[]  ScaleDataTypeStr=Inherit: Inherit via back propagation  ScaleMin=[]  ScaleMax=[]  OffsetDataTypeStr=Inherit: Inherit via back propagation  OffsetMin=[]  OffsetMax=[]  SampleTime=-1
      settings: DataFormat=CT
  block9_ln2 : dlnormalizationlib/Layer Normalization Layer  Layer=quantNet.Layers(81)  OutMin=[]  OutMax=[]  ScaleDataTypeStr=Inherit: Inherit via back propagation  ScaleMin=[]  ScaleMax=[]  OffsetDataTypeStr=Inherit: Inherit via back propagation  OffsetMin=[]  OffsetMax=[]  SampleTime=-1
      settings: DataFormat=CT
  cls_extract : Subsystem
  cls_prepend : Subsystem
  final_ln : dlnormalizationlib/Layer Normalization Layer  Layer=quantNet.Layers(102)  OutMin=[]  OutMax=[]  ScaleDataTypeStr=Inherit: Inherit via back propagation  ScaleMin=[]  ScaleMax=[]  OffsetDataTypeStr=Inherit: Inherit via back propagation  OffsetMin=[]  OffsetMax=[]  SampleTime=-1
      settings: DataFormat=CT
  head : dlconvfclib/Fully Connected Layer  Layer=quantNet.Layers(104)  OutMin=[]  OutMax=[]  OutDataTypeStr=fixdt(1,32,11)  RndMeth=Nearest  WeightsDataTypeStr=fixdt(1,8,9)  WeightsMin=[]  WeightsMax=[]  BiasDataTypeStr=fixdt(1,32,11)  BiasMin=[]  BiasMax=[]  AccumDataTypeStr=fixdt(1,32,11)  AccumMin=[]  AccumMax=[]  InternalRndMeth=Nearest  InternalSaturateOnIntegerOverflow=off  SampleTime=-1
      settings: DataFormat=C
  head_in_cast : DataTypeConversion  OutDataTypeStr=fixdt(1,8,2)  RndMeth=Nearest
  head_out_cast : DataTypeConversion  OutDataTypeStr=single  SaturateOnIntegerOverflow=off  RndMeth=Floor
  patch_conv : dlconvfclib/Convolution 2D Layer  Layer=quantNet.Layers(2)  OutMin=[]  OutMax=[]  OutDataTypeStr=fixdt(1,32,12)  RndMeth=Nearest  SaturateOnIntegerOverflow=on  WeightsDataTypeStr=fixdt(1,8,8)  WeightsMin=[]  WeightsMax=[]  BiasDataTypeStr=fixdt(1,32,12)  BiasMin=[]  BiasMax=[]  AccumDataTypeStr=fixdt(1,32,12)  InternalRndMeth=Nearest  InternalSaturateOnIntegerOverflow=off  SampleTime=-1
      settings: DataFormat=SSC
  patch_conv_in_cast : DataTypeConversion  OutDataTypeStr=fixdt(1,8,4)  RndMeth=Nearest
  patch_conv_out_cast : DataTypeConversion  OutDataTypeStr=fixdt('single')  RndMeth=Nearest
  patch_flatten : Subsystem
  pos_embed : Subsystem
  head_out : Outport
  wires:
  block1_attn/1(out) -> block1_add1/1
  block1_ln1/1 -> block1_attn/1(in)
  block6_ln1/1 -> block6_attn/1(in)
  block5_ln1/1 -> block5_attn/1(in)
  block5_fc1/1 -> block5_fc1_out_cast/1
  patch_flatten/1(out) -> cls_prepend/1(in)
  block5_gelu/1 -> block5_fc2_in_cast/1
  block0_ln2/1 -> block0_fc1_in_cast/1
  block0_gelu/1 -> block0_fc2_in_cast/1
  block8_ln1/1 -> block8_attn/1(in)
  block0_fc1_out_cast/1 -> block0_gelu/1
  block5_fc2/1 -> block5_fc2_out_cast/1
  block2_fc1/1 -> block2_fc1_out_cast/1
  block6_fc1/1 -> block6_fc1_out_cast/1
  patch_conv_in_cast/1 -> patch_conv/1
  block8_fc2/1 -> block8_fc2_out_cast/1
  block1_ln2/1 -> block1_fc1_in_cast/1
  block2_fc2_in_cast/1 -> block2_fc2/1
  block11_fc1_out_cast/1 -> block11_gelu/1
  block9_fc2_out_cast/1 -> block9_add2/1
  block10_fc2_out_cast/1 -> block10_add2/1
  block1_fc2_in_cast/1 -> block1_fc2/1
  block10_ln2/1 -> block10_fc1_in_cast/1
  head_out_cast/1 -> head_out/1
  patch_conv_out_cast/1 -> patch_flatten/1(in)
  head/1 -> head_out_cast/1
  block3_attn/1(out) -> block3_add1/1
  head_in_cast/1 -> head/1
  block7_fc1_in_cast/1 -> block7_fc1/1
  block6_gelu/1 -> block6_fc2_in_cast/1
  block8_ln2/1 -> block8_fc1_in_cast/1
  block11_fc2_out_cast/1 -> block11_add2/1
  block7_fc2_out_cast/1 -> block7_add2/1
  block7_fc2/1 -> block7_fc2_out_cast/1
  block9_fc1_in_cast/1 -> block9_fc1/1
  block9_ln2/1 -> block9_fc1_in_cast/1
  block7_attn/1(out) -> block7_add1/1
  block3_fc1/1 -> block3_fc1_out_cast/1
  block2_gelu/1 -> block2_fc2_in_cast/1
  block5_fc1_out_cast/1 -> block5_gelu/1
  block2_ln1/1 -> block2_attn/1(in)
  block5_fc2_in_cast/1 -> block5_fc2/1
  block8_fc2_in_cast/1 -> block8_fc2/1
  block9_add2/1 -> block10_ln1/1, block10_add1/2
  patch_conv/1 -> patch_conv_out_cast/1
  block2_fc1_in_cast/1 -> block2_fc1/1
  block10_fc1/1 -> block10_fc1_out_cast/1
  block9_fc1/1 -> block9_fc1_out_cast/1
  block4_fc1/1 -> block4_fc1_out_cast/1
  block10_fc2/1 -> block10_fc2_out_cast/1
  block4_ln2/1 -> block4_fc1_in_cast/1
  block3_fc2/1 -> block3_fc2_out_cast/1
  block3_fc1_in_cast/1 -> block3_fc1/1
  block3_ln2/1 -> block3_fc1_in_cast/1
  block4_ln1/1 -> block4_attn/1(in)
  block11_add2/1 -> final_ln/1
  block10_gelu/1 -> block10_fc2_in_cast/1
  block3_gelu/1 -> block3_fc2_in_cast/1
  block9_fc2/1 -> block9_fc2_out_cast/1
  block6_fc2_out_cast/1 -> block6_add2/1
  block10_attn/1(out) -> block10_add1/1
  block7_add1/1 -> block7_add2/2, block7_ln2/1
  block9_attn/1(out) -> block9_add1/1
  block1_add1/1 -> block1_ln2/1, block1_add2/2
  block0_ln1/1 -> block0_attn/1(in)
  block5_ln2/1 -> block5_fc1_in_cast/1
  block10_fc1_out_cast/1 -> block10_gelu/1
  block11_attn/1(out) -> block11_add1/1
  block4_fc1_out_cast/1 -> block4_gelu/1
  block4_fc2_out_cast/1 -> block4_add2/1
  block1_fc2_out_cast/1 -> block1_add2/1
  block8_fc1/1 -> block8_fc1_out_cast/1
  block5_fc1_in_cast/1 -> block5_fc1/1
  block6_add2/1 -> block7_ln1/1, block7_add1/2
  block0_add2/1 -> block1_ln1/1, block1_add1/2
  block0_fc1_in_cast/1 -> block0_fc1/1
  block3_fc1_out_cast/1 -> block3_gelu/1
  block1_fc1/1 -> block1_fc1_out_cast/1
  block7_add2/1 -> block8_ln1/1, block8_add1/2
  block2_fc2/1 -> block2_fc2_out_cast/1
  block8_attn/1(out) -> block8_add1/1
  block8_gelu/1 -> block8_fc2_in_cast/1
  block1_fc2/1 -> block1_fc2_out_cast/1
  block1_gelu/1 -> block1_fc2_in_cast/1
  block0_fc1/1 -> block0_fc1_out_cast/1
  block4_add2/1 -> block5_ln1/1, block5_add1/2
  block1_add2/1 -> block2_add1/2, block2_ln1/1
  block6_fc2/1 -> block6_fc2_out_cast/1
  block10_add2/1 -> block11_ln1/1, block11_add1/2
  block8_fc2_out_cast/1 -> block8_add2/1
  block7_gelu/1 -> block7_fc2_in_cast/1
  block4_fc2/1 -> block4_fc2_out_cast/1
  block6_ln2/1 -> block6_fc1_in_cast/1
  pos_embed/1(out) -> block0_ln1/1, block0_add1/2
  block9_fc2_in_cast/1 -> block9_fc2/1
  input/1 -> patch_conv_in_cast/1
  block11_ln1/1 -> block11_attn/1(in)
  block3_ln1/1 -> block3_attn/1(in)
  block0_fc2/1 -> block0_fc2_out_cast/1
  block2_fc2_out_cast/1 -> block2_add2/1
  block7_fc1_out_cast/1 -> block7_gelu/1
  block11_fc1_in_cast/1 -> block11_fc1/1
  block4_fc2_in_cast/1 -> block4_fc2/1
  block6_fc1_out_cast/1 -> block6_gelu/1
  block7_ln2/1 -> block7_fc1_in_cast/1
  cls_extract/1(out) -> head_in_cast/1
  block9_gelu/1 -> block9_fc2_in_cast/1
  block6_attn/1(out) -> block6_add1/1
  final_ln/1 -> cls_extract/1(in)
  block4_gelu/1 -> block4_fc2_in_cast/1
  block9_add1/1 -> block9_add2/2, block9_ln2/1
  block8_add1/1 -> block8_ln2/1, block8_add2/2
  block4_add1/1 -> block4_ln2/1, block4_add2/2
  block0_fc2_out_cast/1 -> block0_add2/1
  block10_fc1_in_cast/1 -> block10_fc1/1
  block1_fc1_in_cast/1 -> block1_fc1/1
  block0_fc2_in_cast/1 -> block0_fc2/1
  block5_add1/1 -> block5_ln2/1, block5_add2/2
  block8_fc1_out_cast/1 -> block8_gelu/1
  block11_fc2_in_cast/1 -> block11_fc2/1
  block5_fc2_out_cast/1 -> block5_add2/1
  block9_fc1_out_cast/1 -> block9_gelu/1
  block11_fc1/1 -> block11_fc1_out_cast/1
  block10_add1/1 -> block10_ln2/1, block10_add2/2
  block4_attn/1(out) -> block4_add1/1
  block4_fc1_in_cast/1 -> block4_fc1/1
  block9_ln1/1 -> block9_attn/1(in)
  block3_add1/1 -> block3_ln2/1, block3_add2/2
  block2_add2/1 -> block3_ln1/1, block3_add1/2
  block3_fc2_in_cast/1 -> block3_fc2/1
  block5_attn/1(out) -> block5_add1/1
  block2_fc1_out_cast/1 -> block2_gelu/1
  block7_fc2_in_cast/1 -> block7_fc2/1
  block7_ln1/1 -> block7_attn/1(in)
  block3_fc2_out_cast/1 -> block3_add2/1
  block11_ln2/1 -> block11_fc1_in_cast/1
  block11_gelu/1 -> block11_fc2_in_cast/1
  block6_fc1_in_cast/1 -> block6_fc1/1
  block7_fc1/1 -> block7_fc1_out_cast/1
  cls_prepend/1(out) -> pos_embed/1(in)
  block1_fc1_out_cast/1 -> block1_gelu/1
  block10_ln1/1 -> block10_attn/1(in)
  block0_attn/1(out) -> block0_add1/1
  block11_add1/1 -> block11_ln2/1, block11_add2/2
  block0_add1/1 -> block0_ln2/1, block0_add2/2
  block8_fc1_in_cast/1 -> block8_fc1/1
  block10_fc2_in_cast/1 -> block10_fc2/1
  block6_add1/1 -> block6_ln2/1, block6_add2/2
  block2_ln2/1 -> block2_fc1_in_cast/1
  block2_add1/1 -> block2_ln2/1, block2_add2/2
  block3_add2/1 -> block4_ln1/1, block4_add1/2
  block6_fc2_in_cast/1 -> block6_fc2/1
  block2_attn/1(out) -> block2_add1/1
  block11_fc2/1 -> block11_fc2_out_cast/1
  block5_add2/1 -> block6_add1/2, block6_ln1/1
  block8_add2/1 -> block9_ln1/1, block9_add1/2

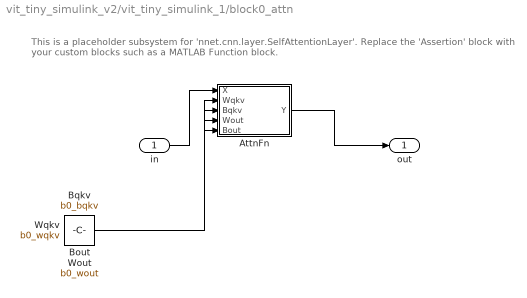
== system vit_tiny_simulink_v2/vit_tiny_simulink_1/block0_attn ==
  note: This is a placeholder subsystem for 'nnet.cnn.layer.SelfAttentionLayer'. Replace the 'Assertion' block with your custom blocks such as a MATLAB Function block.
  in : Inport
  AttnFn : MATLAB Function  TreatAsAtomicUnit=on
  Bout : Constant  Value=b0_bout  OutDataTypeStr=single  SampleTime=-1
  Bqkv : Constant  Value=b0_bqkv  OutDataTypeStr=single  SampleTime=-1
  Wout : Constant  Value=b0_wout  OutDataTypeStr=single  SampleTime=-1
  Wqkv : Constant  Value=b0_wqkv  OutDataTypeStr=single  SampleTime=-1
  out : Outport
  wires:
  in/1 -> AttnFn/1(X)
  Wqkv/1 -> AttnFn/2(Wqkv)
  Bqkv/1 -> AttnFn/3(Bqkv)
  Wout/1 -> AttnFn/4(Wout)
  Bout/1 -> AttnFn/5(Bout)
  AttnFn/1(Y) -> out/1
